simulink model "ospfb_2x_64kc_8i_16o"  (R2021a)
settings: Solver=VariableStepAuto  StartTime=0.0  StopTime=2304  RelTol=1e-3
undefined symbols (not defined in the file or in its folder): pfb_coeff_gen_calc
libraries: xbsIndex_r4 x37193, casper_library_misc x226, casper_library_delays x184
(part 199 of 313)

== system ospfb_2x_64kc_8i_16o/fft2/fft_direct/butterfly3_6/twiddle/coeff_gen/cosin ==
  theta : Inport
  misci : Inport  Port=2
  Constant4 : xbsIndex_r4/Constant  const=0  gui_display_data_type=Fixed-point  arith_type=Unsigned  n_bits=1  bin_pt=0  preci_type=Single  exp_width=8  frac_width=24  explicit_period=on  period=1  equ=P=C  opselect=C  inp2=PCIN>>17  opr=+  inp1=P  carry=CIN  dbl_ovrd=off  block_type=constant
  Constant5 : xbsIndex_r4/Constant  const=0  gui_display_data_type=Fixed-point  arith_type=Unsigned  n_bits=1  bin_pt=0  preci_type=Single  exp_width=8  frac_width=24  explicit_period=on  period=1  equ=P=C  opselect=C  inp2=PCIN>>17  opr=+  inp1=P  carry=CIN  dbl_ovrd=off  block_type=constant
  Delay : xbsIndex_r4/Delay  rst=off  infoeditControl=Selection of Reset will increase slice count due to use of real FFs and instead of SRLs  en=off  latency=bram_latency  reg_retiming=on  dbl_ovrd=off  block_type=delay
  Delay10 : xbsIndex_r4/Delay  rst=off  infoeditControl=Selection of Reset will increase slice count due to use of real FFs and instead of SRLs  en=off  latency=bram_latency  reg_retiming=on  dbl_ovrd=off  block_type=delay
  Delay8 : xbsIndex_r4/Delay  rst=off  infoeditControl=Selection of Reset will increase slice count due to use of real FFs and instead of SRLs  en=off  latency=bram_latency  reg_retiming=on  dbl_ovrd=off  block_type=delay
  ROM : xbsIndex_r4/ROM  depth=2^(12)  initVector=[13149021945 13145876212 13142992624 13139846891 13136963302 13133817569 13130671836 13127788248 13124642515 13121758926 13118613193 13115467460 13112583872 13109438139 13106554550 13103408817 13100263084 13097379496 13094233763 13091088030 13088204441 13085058709 13082175120 13079029387 13075883654 13073000065 13069854333 13066708600 13063825011 13060679278 13057795689 13054649957 13051504224 13048620635 13045474902 13042329170 13039445581 13036299848 13033416259 13030270527 13027124794 13024241205 13021095472 13017949740 13015066151 13011920418 13009036829 13005891096 13002745364 12999861775 12996716042 12993570309 12990686721 12987540988 12984395255 12981511666 12978365934 12975482345 12972336612 12969190879 12966307291 12963161558 12960015825 12957132237 12953986504 12950840771 12947957182 12944811450 12941927861 12938782128 12935636395 12932752807 12929607074 12926461341 12923577752 12920432020 12917286287 12914402698 12911256966 12908111233 12905227644 12902081911 12899198323 12896052590 12892906857 12890023269 12886877536 12883731803 12880848214 12877702482 12874556749 12871673160 12868527428 12865381695 12862498106 12859352373 12856206641 12853323052 12850177319 12847031587 12844147998 12841002265 12838118677 12834972944 12831827211 12828943622 12825797890 12822652157 12819768568 12816622836 12813477103 12810593514 12807447782 12804302049 12801418460 12798272728 12795126995 12792243406 12789097674 12785951941 12783068352 12779922620 12776776887 12773893298 12770747566 12767601833 12764718244 12761572512 12758426779 12755543190 12752397458 12749251725 12746368136 12743222404 12740076671 12737193082 12734047350 12730901617 12728018028 12724872296 12721726563 12718842974 12715697242 12712551509 12709667920 12706522188 12703376455 12700492866 12697347134 12694201401 12691055668 12688172080 12685026347 12681880615 12678997026 12675851293 12672705561 12669821972 12666676239 12663530507 12660646918 12657501185 12654355453 12651471864 12648326132 12645180399 12642296810 12639151078 12636005345 12633121756 12629976024 12626830291 12623684559 12620800970 12617655237 12614509505 12611625916 12608480184 12605334451 12602450862 12599305130 12596159397 12593275809 12590130076 12586984343 12583838611 12580955022 12577809289 12574663557 12571779968 12568634236 12565488503 12562604915 12559459182 12556313449 12553429861 12550284128 12547138396 12543992663 12541109074 12537963342 12534817609 12531934021 12528788288 12525642555 12522758967 12519613234 12516467502 12513321769 12510438181 12507292448 12504146715 12501263127 12498117394 12494971662 12491825929 12488942341 12485796608 12482650875 12479767287 12476621554 12473475822 12470592233 12467446501 12464300768 12461155036 12458271447 12455125714 12451979982 12449096393 12445950661 12442804928 12439659196 12436775607 12433629875 12430484142 12427600553 12424454821 12421309088 12418163356 12415279767 12412134035 12408988302 12406104714 12402958981 12399813249 12396667516 12393783928 12390638195 12387492462 12384608874 12381463141 12378317409 12375171676 12372288088 12369142355 12365996623 12362850890 12359967302 12356821569 12353675837 12350792248 12347646516 12344500783 12341355051 12338471462 12335325730 12332179997 12329034265 12326150676 12323004944 12319859211 12316975623 12313829890 12310684158 12307538425 12304654837 12301509104 12298363372 12295217639 12292334051 12289188318 12286042586 12282896853 12280013265 12276867532 12273721800 12270838211 12267692479 12264546746 12261401014 12258517425 12255371693 12252225960 12249080228 12246196639 12243050907 12239905174 12236759442 12233875853 12230730121 12227584388 12224438656 12221555067 12218409335 12215263603 12212117870 12209234282 12206088549 12202942817 12199797084 12196913496 12193767763 12190622031 12187476298 12184592710 12181446977 12178301245 12175155513 12172271924 12169126192 12165980459 12162834727 12159951138 12156805406 12153659673 12150513941 12147630353 12144484620 12141338888 12138193155 12135309567 12132163834 ...(+41741 chars)]  distributed_mem=Block RAM  rst=off  init_reg=0  en=off  latency=bram_latency  gui_display_data_type=Fixed-point  arith_type=Unsigned  n_bits=n_bits*2  bin_pt=0  preci_type=Single  exp_width=8  frac_width=24  optimize=Speed  use_rpm=on  dbl_ovrd=off  simulation_type=fast_sim  block_type=sprom
  Terminator : Terminator
  Terminator1 : Terminator
  Terminator4 : Terminator
  add_convert0 : Subsystem
  add_convert1 : Subsystem
  assert : xbsIndex_r4/Assert  assert_type=on  type_source=Explicitly  gui_display_data_type=Fixed-point  arith_type=Unsigned  n_bits=12  bin_pt=0  preci_type=Single  exp_width=8  frac_width=24  assert_rate=off  rate_source=Explicitly  period=1  output_port=on  block_type=assert
  c_to_ri : casper_library_misc/c_to_ri  n_bits=n_bits  bin_pt=bin_pt
      settings: SourceProductName=CASPER DSP Blockset
  invert0 : Subsystem  mask{n_bits=n_bits, bin_pt=bin_pt, neg_latency=neg_latency, mux_latency=mux_latency}
      mask prompts: n_bits "bit width"; bin_pt "binary point"; neg_latency "negate latency"; mux_latency "mux latency"
  invert1 : Subsystem  mask{n_bits=n_bits, bin_pt=bin_pt, neg_latency=neg_latency, mux_latency=mux_latency}
      mask prompts: n_bits "bit width"; bin_pt "binary point"; neg_latency "negate latency"; mux_latency "mux latency"
  cos : Outport
  -sin : Outport  Port=2
  misco : Outport  Port=3
  wires:
  theta/1 -> assert/1
  assert/1 -> add_convert0/1(theta), add_convert1/1(theta)
  Constant4/1 -> add_convert0/2(misci)
  add_convert0/3(misco) -> Terminator/1
  misci/1 -> add_convert1/2(misci)
  add_convert1/3(misco) -> Delay/1
  add_convert0/2(add) -> ROM/1
  add_convert1/2(add) -> Terminator4/1
  ROM/1 -> c_to_ri/1
  add_convert1/1(negate) -> Delay8/1
  add_convert0/1(negate) -> Delay10/1
  Delay10/1 -> invert0/1(negate)
  Constant5/1 -> invert0/3(misci)
  c_to_ri/1 -> invert0/2(in)
  Delay8/1 -> invert1/1(negate)
  c_to_ri/2 -> invert1/2(in)
  invert0/2(misco) -> Terminator1/1
  Delay/1 -> invert1/3(misci)
  invert0/1(out) -> cos/1
  invert1/1(out) -> -sin/1
  invert1/2(misco) -> misco/1
